simulink model "CCMHfaMotor"  (R2022b) (continued)
== system CCMHfaMotor == (continued)
  HfaActr14BlckSts_HfaActr14BlckSts : Inport  Port=341  OutDataTypeStr=Enum: VentnActr01BlckSts  PortDimensions=1
  HfaActr14BusAdr_HfaActr14BusAdr : Inport  Port=342  OutDataTypeStr=UInt8  PortDimensions=1
  HfaActr14CoilSts_HfaActr14CoilSts : Inport  Port=343  OutDataTypeStr=Enum: VentnMotUrgcUnlck  PortDimensions=1
  HfaActr14DrvDir_HfaActr14DrvDir : Inport  Port=344  OutDataTypeStr=Enum: VentnActr01DrvDir  PortDimensions=1
  HfaActr14ElecErr_HfaActr14ElecErr : Inport  Port=345  OutDataTypeStr=Enum: VentnActr01ElecErr  PortDimensions=1
  HfaActr14Mode_HfaActr14Mode : Inport  Port=346  OutDataTypeStr=Enum: VentnMotMode  PortDimensions=1
  HfaActr14MovDir_HfaActr14MovDir : Inport  Port=347  OutDataTypeStr=Enum: VentnMotDir  PortDimensions=1
  HfaActr14OvrTempErr_HfaActr14OvrTempErr : Inport  Port=348  OutDataTypeStr=Enum: VentnActr01OvrTempErr  PortDimensions=1
  HfaActr14PsonSts_HfaActr14PsonSts : Inport  Port=349  OutDataTypeStr=Enum: VentnMotPsonAvl  PortDimensions=1
  HfaActr14ResetSts_HfaActr14ResetSts : Inport  Port=350  OutDataTypeStr=Enum: VentnActr01ResetSts  PortDimensions=1
  HfaActr14SpclFct_HfaActr14SpclFct : Inport  Port=351  OutDataTypeStr=Enum: VentnActr01SpclFct  PortDimensions=1
  HfaActr14SpdSts_HfaActr14SpdSts : Inport  Port=352  OutDataTypeStr=Enum: VentnActr01SpdSts  PortDimensions=1
  HfaActr14TrqSts_HfaActr14TrqSts : Inport  Port=353  OutDataTypeStr=Enum: VentnMotUrgcUnlck  PortDimensions=1
  HfaActr14UrgcExist_HfaActr14UrgcExist : Inport  Port=354  OutDataTypeStr=Enum: VentnActr01UrgcExist  PortDimensions=1
  HfaActr14UrgcPson_HfaActr14UrgcPson : Inport  Port=355  OutDataTypeStr=Enum: VentnMotUrgcPson  PortDimensions=1
  HfaActr14UrgcSts_HfaActr14UrgcSts : Inport  Port=356  OutDataTypeStr=Enum: VentnMotUrgcUnlck  PortDimensions=1
  HfaActr14VoltErr_HfaActr14VoltErr : Inport  Port=357  OutDataTypeStr=Enum: VentnActr01VoltErr  PortDimensions=1
  HfaActr15ActPson_HfaActr15ActPson : Inport  Port=358  OutDataTypeStr=UInt16  PortDimensions=1
  HfaActr15ActPson_HfaActr15ActPson_IsUpdated : Inport  Port=359  OutDataTypeStr=boolean  PortDimensions=1
  HfaActr15BlckInd_HfaActr15BlckInd : Inport  Port=360  OutDataTypeStr=Enum: VentnActr01BlckInd  PortDimensions=1
  HfaActr15BlckSts_HfaActr15BlckSts : Inport  Port=361  OutDataTypeStr=Enum: VentnActr01BlckSts  PortDimensions=1
  HfaActr15BusAdr_HfaActr15BusAdr : Inport  Port=362  OutDataTypeStr=UInt8  PortDimensions=1
  HfaActr15CoilSts_HfaActr15CoilSts : Inport  Port=363  OutDataTypeStr=Enum: VentnMotUrgcUnlck  PortDimensions=1
  HfaActr15DrvDir_HfaActr15DrvDir : Inport  Port=364  OutDataTypeStr=Enum: VentnActr01DrvDir  PortDimensions=1
  HfaActr15ElecErr_HfaActr15ElecErr : Inport  Port=365  OutDataTypeStr=Enum: VentnActr01ElecErr  PortDimensions=1
  HfaActr15Mode_HfaActr15Mode : Inport  Port=366  OutDataTypeStr=Enum: VentnMotMode  PortDimensions=1
  HfaActr15MovDir_HfaActr15MovDir : Inport  Port=367  OutDataTypeStr=Enum: VentnMotDir  PortDimensions=1
  HfaActr15OvrTempErr_HfaActr15OvrTempErr : Inport  Port=368  OutDataTypeStr=Enum: VentnActr01OvrTempErr  PortDimensions=1
  HfaActr15PsonSts_HfaActr15PsonSts : Inport  Port=369  OutDataTypeStr=Enum: VentnMotPsonAvl  PortDimensions=1
  HfaActr15ResetSts_HfaActr15ResetSts : Inport  Port=370  OutDataTypeStr=Enum: VentnActr01ResetSts  PortDimensions=1
  HfaActr15SpclFct_HfaActr15SpclFct : Inport  Port=371  OutDataTypeStr=Enum: VentnActr01SpclFct  PortDimensions=1
  HfaActr15SpdSts_HfaActr15SpdSts : Inport  Port=372  OutDataTypeStr=Enum: VentnActr01SpdSts  PortDimensions=1
  HfaActr15TrqSts_HfaActr15TrqSts : Inport  Port=373  OutDataTypeStr=Enum: VentnMotUrgcUnlck  PortDimensions=1
  HfaActr15UrgcExist_HfaActr15UrgcExist : Inport  Port=374  OutDataTypeStr=Enum: VentnActr01UrgcExist  PortDimensions=1
  HfaActr15UrgcPson_HfaActr15UrgcPson : Inport  Port=375  OutDataTypeStr=Enum: VentnMotUrgcPson  PortDimensions=1
  HfaActr15UrgcSts_HfaActr15UrgcSts : Inport  Port=376  OutDataTypeStr=Enum: VentnMotUrgcUnlck  PortDimensions=1
  HfaActr15VoltErr_HfaActr15VoltErr : Inport  Port=377  OutDataTypeStr=Enum: VentnActr01VoltErr  PortDimensions=1
  HvacCfgForClimaCtrl_HvacCfgForAQSTypes : Inport  Port=378  OutDataTypeStr=UInt8  PortDimensions=1
  HvacCfgForClimaCtrl_HvacCfgForHvacLowSideRETXVESts : Inport  Port=379  OutDataTypeStr=UInt8  PortDimensions=1
  HvacCfgForClimaCtrl_HvacCfgForHvacReBlowerType : Inport  Port=380  OutDataTypeStr=UInt8  PortDimensions=1
  HvacCfgForClimaCtrl_HvacCfgForHvacReVentPstn : Inport  Port=381  OutDataTypeStr=UInt8  PortDimensions=1
  HvacCfgForClimaCtrl_HvacCfgForHvacSnsrRCmptmtESts : Inport  Port=382  OutDataTypeStr=UInt8  PortDimensions=1
  HvacCfgForClimaCtrl_HvacCfgForHvacSnsrReHexESts : Inport  Port=383  OutDataTypeStr=UInt8  PortDimensions=1
  Lin1ActvReady_Lin1ActvReady : Inport  Port=384  OutDataTypeStr=UInt8  PortDimensions=1
  Lin2ActvReady_Lin2ActvReady : Inport  Port=385  OutDataTypeStr=UInt8  PortDimensions=1
  ReHvacActrActPson_ReHvacActrActPson : Inport  Port=386  OutDataTypeStr=UInt16  PortDimensions=1
  ReHvacActrActPson_ReHvacActrActPson_IsUpdated : Inport  Port=387  OutDataTypeStr=boolean  PortDimensions=1
  ReHvacActrBlckInd_ReHvacActrBlckInd : Inport  Port=388  OutDataTypeStr=Enum: VentnActr01BlckInd  PortDimensions=1
  ReHvacActrBlckSts_ReHvacActrBlckSts : Inport  Port=389  OutDataTypeStr=Enum: VentnActr01BlckSts  PortDimensions=1
  ReHvacActrBusAdr_ReHvacActrBusAdr : Inport  Port=390  OutDataTypeStr=UInt8  PortDimensions=1
  ReHvacActrCoilSts_ReHvacActrCoilSts : Inport  Port=391  OutDataTypeStr=Enum: VentnMotUrgcUnlck  PortDimensions=1
  ReHvacActrDrvDir_ReHvacActrDrvDir : Inport  Port=392  OutDataTypeStr=Enum: VentnActr01DrvDir  PortDimensions=1
  ReHvacActrElecErr_ReHvacActrElecErr : Inport  Port=393  OutDataTypeStr=Enum: VentnActr01ElecErr  PortDimensions=1
  ReHvacActrMode_ReHvacActrMode : Inport  Port=394  OutDataTypeStr=Enum: VentnMotMode  PortDimensions=1
  ReHvacActrMovDir_ReHvacActrMovDir : Inport  Port=395  OutDataTypeStr=Enum: VentnMotDir  PortDimensions=1
  ReHvacActrOvrTempErr_ReHvacActrOvrTempErr : Inport  Port=396  OutDataTypeStr=Enum: VentnActr01OvrTempErr  PortDimensions=1
  ReHvacActrPsonSts_ReHvacActrPsonSts : Inport  Port=397  OutDataTypeStr=Enum: VentnMotPsonAvl  PortDimensions=1
  ReHvacActrResetSts_ReHvacActrResetSts : Inport  Port=398  OutDataTypeStr=Enum: VentnActr01ResetSts  PortDimensions=1
  ReHvacActrSpclFct_ReHvacActrSpclFct : Inport  Port=399  OutDataTypeStr=Enum: VentnActr01SpclFct  PortDimensions=1
  ReHvacActrSpdSts_ReHvacActrSpdSts : Inport  Port=400  OutDataTypeStr=Enum: VentnActr01SpdSts  PortDimensions=1
  ReHvacActrTrqSts_ReHvacActrTrqSts : Inport  Port=401  OutDataTypeStr=Enum: VentnMotUrgcUnlck  PortDimensions=1
  ReHvacActrUrgcExist_ReHvacActrUrgcExist : Inport  Port=402  OutDataTypeStr=Enum: VentnActr01UrgcExist  PortDimensions=1
  ReHvacActrUrgcPson_ReHvacActrUrgcPson : Inport  Port=403  OutDataTypeStr=Enum: VentnMotUrgcPson  PortDimensions=1
  ReHvacActrUrgcSts_ReHvacActrUrgcSts : Inport  Port=404  OutDataTypeStr=Enum: VentnMotUrgcUnlck  PortDimensions=1
  ReHvacActrVoltErr_ReHvacActrVoltErr : Inport  Port=405  OutDataTypeStr=Enum: VentnActr01VoltErr  PortDimensions=1
  SysServCarCfgSts_SysServCCPBGMNum : Inport  Port=406  OutDataTypeStr=UInt8  PortDimensions=1
  SysServCarCfgSts_SysServCCPLin2AQSSts : Inport  Port=407  OutDataTypeStr=Boolean  PortDimensions=1
  SysServCarCfgSts_SysServDUBlowerPwmSignTypeSts : Inport  Port=408  OutDataTypeStr=UInt8  PortDimensions=1
  SysServCarCfgSts_SysServDUDCFLMdeSts : Inport  Port=409  OutDataTypeStr=UInt8  PortDimensions=1
  SysServCarCfgSts_SysServDULin4HFAFStsH : Inport  Port=410  OutDataTypeStr=UInt32  PortDimensions=1
  SysServCarCfgSts_SysServDUReBlowerPwmSignSts : Inport  Port=411  OutDataTypeStr=UInt8  PortDimensions=1
  SysServCarCfgSts_SysServDUReBlowerPwmSts : Inport  Port=412  OutDataTypeStr=UInt8  PortDimensions=1
  SysServCarCfgSts_SysServFloatingMotorSts : Inport  Port=413  OutDataTypeStr=UInt8  PortDimensions=1
  SysServCarCfgSts_SysServFrntHEXSnsrPosn : Inport  Port=414  OutDataTypeStr=UInt8  PortDimensions=1
  SysServCarCfgSts_SysServLowSideRETXVSts : Inport  Port=415  OutDataTypeStr=UInt8  PortDimensions=1
  SysServCarCfgSts_SysServReHEXSnsrPosn : Inport  Port=416  OutDataTypeStr=UInt8  PortDimensions=1
  SysServCarCfgSts_SysServRHFASts : Inport  Port=417  OutDataTypeStr=UInt8  PortDimensions=1
  SysServCarCfgSts_SysServSnsrForFCmptmtTType : Inport  Port=418  OutDataTypeStr=UInt8  PortDimensions=1
  WtrActrLeActPson_WtrActrLeActPson : Inport  Port=419  OutDataTypeStr=UInt16  PortDimensions=1
  WtrActrLeBlckInd_WtrActrLeBlckInd : Inport  Port=420  OutDataTypeStr=Enum: VentnActr01BlckInd  PortDimensions=1
  WtrActrLeBlckSts_WtrActrLeBlckSts : Inport  Port=421  OutDataTypeStr=Enum: VentnActr01BlckSts  PortDimensions=1
  WtrActrLeBusAdr_WtrActrLeBusAdr : Inport  Port=422  OutDataTypeStr=UInt8  PortDimensions=1
  WtrActrLeCoilSts_WtrActrLeCoilSts : Inport  Port=423  OutDataTypeStr=Enum: VentnMotUrgcUnlck  PortDimensions=1
  WtrActrLeDir_WtrActrLeDir : Inport  Port=424  OutDataTypeStr=Enum: VentnActr01DrvDir  PortDimensions=1
  WtrActrLeElecErr_WtrActrLeElecErr : Inport  Port=425  OutDataTypeStr=Enum: VentnActr01ElecErr  PortDimensions=1
  WtrActrLeMode_WtrActrLeMode : Inport  Port=426  OutDataTypeStr=Enum: VentnMotMode  PortDimensions=1
  WtrActrLeMovDir_WtrActrLeMovDir : Inport  Port=427  OutDataTypeStr=Enum: VentnMotDir  PortDimensions=1
  WtrActrLeOvrTempErr_WtrActrLeOvrTempErr : Inport  Port=428  OutDataTypeStr=Enum: VentnActr01OvrTempErr  PortDimensions=1
  WtrActrLePsonSts_WtrActrLePsonSts : Inport  Port=429  OutDataTypeStr=Enum: VentnMotPsonAvl  PortDimensions=1
  WtrActrLeResetSts_WtrActrLeResetSts : Inport  Port=430  OutDataTypeStr=Enum: VentnActr01ResetSts  PortDimensions=1
  WtrActrLeSpclFct_WtrActrLeSpclFct : Inport  Port=431  OutDataTypeStr=Enum: VentnActr01SpclFct  PortDimensions=1
  WtrActrLeSpdSts_WtrActrLeSpdSts : Inport  Port=432  OutDataTypeStr=Enum: VentnActr01SpdSts  PortDimensions=1
  WtrActrLeTrqSts_WtrActrLeTrqSts : Inport  Port=433  OutDataTypeStr=Enum: VentnMotUrgcUnlck  PortDimensions=1
  WtrActrLeUrgcExist_WtrActrLeUrgcExist : Inport  Port=434  OutDataTypeStr=Enum: VentnActr01UrgcExist  PortDimensions=1
  WtrActrLeUrgcPson_WtrActrLeUrgcPson : Inport  Port=435  OutDataTypeStr=Enum: VentnMotUrgcPson  PortDimensions=1
  WtrActrLeUrgcSts_WtrActrLeUrgcSts : Inport  Port=436  OutDataTypeStr=Enum: VentnMotUrgcUnlck  PortDimensions=1
  WtrActrLeVoltErr_WtrActrLeVoltErr : Inport  Port=437  OutDataTypeStr=Enum: VentnActr01VoltErr  PortDimensions=1
  WtrActrRiActPson_WtrActrRiActPson : Inport  Port=438  OutDataTypeStr=UInt16  PortDimensions=1
  WtrActrRiBlckInd_WtrActrRiBlckInd : Inport  Port=439  OutDataTypeStr=Enum: VentnActr01BlckInd  PortDimensions=1
  WtrActrRiBlckSts_WtrActrRiBlckSts : Inport  Port=440  OutDataTypeStr=Enum: VentnActr01BlckSts  PortDimensions=1
  WtrActrRiBusAdr_WtrActrRiBusAdr : Inport  Port=441  OutDataTypeStr=UInt8  PortDimensions=1
  WtrActrRiCoilSts_WtrActrRiCoilSts : Inport  Port=442  OutDataTypeStr=Enum: VentnMotUrgcUnlck  PortDimensions=1
  WtrActrRiDir_WtrActrRiDir : Inport  Port=443  OutDataTypeStr=Enum: VentnActr01DrvDir  PortDimensions=1
  WtrActrRiElecErr_WtrActrRiElecErr : Inport  Port=444  OutDataTypeStr=Enum: VentnActr01ElecErr  PortDimensions=1
  WtrActrRiMode_WtrActrRiMode : Inport  Port=445  OutDataTypeStr=Enum: VentnMotMode  PortDimensions=1
  WtrActrRiMovDir_WtrActrRiMovDir : Inport  Port=446  OutDataTypeStr=Enum: VentnMotDir  PortDimensions=1
  WtrActrRiOvrTempErr_WtrActrRiOvrTempErr : Inport  Port=447  OutDataTypeStr=Enum: VentnActr01OvrTempErr  PortDimensions=1
  WtrActrRiPsonSts_WtrActrRiPsonSts : Inport  Port=448  OutDataTypeStr=Enum: VentnMotPsonAvl  PortDimensions=1
  WtrActrRiResetSts_WtrActrRiResetSts : Inport  Port=449  OutDataTypeStr=Enum: VentnActr01ResetSts  PortDimensions=1
  WtrActrRiSpclFct_WtrActrRiSpclFct : Inport  Port=450  OutDataTypeStr=Enum: VentnActr01SpclFct  PortDimensions=1
  WtrActrRiSpdSts_WtrActrRiSpdSts : Inport  Port=451  OutDataTypeStr=Enum: VentnActr01SpdSts  PortDimensions=1
  WtrActrRiTrqSts_WtrActrRiTrqSts : Inport  Port=452  OutDataTypeStr=Enum: VentnMotUrgcUnlck  PortDimensions=1
  WtrActrRiUrgcExist_WtrActrRiUrgcExist : Inport  Port=453  OutDataTypeStr=Enum: VentnActr01UrgcExist  PortDimensions=1
  WtrActrRiUrgcPson_WtrActrRiUrgcPson : Inport  Port=454  OutDataTypeStr=Enum: VentnMotUrgcPson  PortDimensions=1
  WtrActrRiUrgcSts_WtrActrRiUrgcSts : Inport  Port=455  OutDataTypeStr=Enum: VentnMotUrgcUnlck  PortDimensions=1
  WtrActrRiVoltErr_WtrActrRiVoltErr : Inport  Port=456  OutDataTypeStr=Enum: VentnActr01VoltErr  PortDimensions=1
  BswLifeCycle_LifeCycle : Inport  Port=457  OutDataTypeStr=Enum: CCMLifeCycleModeGroup  PortDimensions=1
  CCMHfaMotor_HfaMotMode_sys : Subsystem
  CCMHfaMotor_ReHvacMotMode_sys : Subsystem
  CCMHfaMotor_WtrMotMode_sys : Subsystem
  HfaCaliService : Subsystem
  HfaDtcService : Subsystem
  HfaIOCtrlService : Subsystem
  HfaMotor_50msRunnable_sys : Subsystem
  HfaMotor_Init : Subsystem
  HfaMotor_Runnable_sys : Subsystem
  HfaNvmService : Subsystem
  HfaParaService : Subsystem
  HfaRIDService : Subsystem
  NvMBlockHfaMtrCalData : DataStoreMemory  DataStoreName=NvMBlockHfaMtrCalData  InitialValue=NvMBlockHfaMtrCalDataInit  ShowAdditionalParam=off  Dimensions=200  StateMustResolveToSignalObject=on
  ActCfrAddrFinalAddr_ActCfrAddrFinalAddr : Outport  OutDataTypeStr=UInt8  PortDimensions=1
  ActCfrAddrGroup_ActCfrAddrGroup : Outport  Port=2  OutDataTypeStr=Enum: ActCfrGroup  PortDimensions=1
  ActCfrAddrItem_ActCfrAddrItem : Outport  Port=3  OutDataTypeStr=Uint5  PortDimensions=1
  ActCfrIniCPos_ActCfrIniCPos : Outport  Port=4  OutDataTypeStr=UInt15  PortDimensions=1
  ActCfrIniGroup_ActCfrIniGroup : Outport  Port=5  OutDataTypeStr=Enum: ActCfrGroup  PortDimensions=1
  ActCfrIniItem_ActCfrIniItem : Outport  Port=6  OutDataTypeStr=Uint5  PortDimensions=1
  ActCfrIniResvd_ActCfrIniResvd : Outport  Port=7  OutDataTypeStr=UInt8  PortDimensions=1
  ActCfrModGroup_ActCfrModGroup : Outport  Port=8  OutDataTypeStr=Enum: ActCfrGroup  PortDimensions=1
  ActCfrModItem_ActCfrModItem : Outport  Port=9  OutDataTypeStr=Uint5  PortDimensions=1
  ActCfrModNewMod_ActCfrModNewMod : Outport  Port=10  OutDataTypeStr=Enum: ActCfrMod  PortDimensions=1
  ActCfrModResvd1_ActCfrModResvd1 : Outport  Port=11  OutDataTypeStr=UInt8  PortDimensions=1
  ActCfrModResvd2_ActCfrModResvd2 : Outport  Port=12  OutDataTypeStr=UInt8  PortDimensions=1
  ActCfrPrgDiret_ActCfrPrgDiret : Outport  Port=13  OutDataTypeStr=Enum: ActCfrPrgDir  PortDimensions=1
  ActCfrPrgGroup_ActCfrPrgGroup : Outport  Port=14  OutDataTypeStr=Enum: ActCfrGroup  PortDimensions=1
  ActCfrPrgItem_ActCfrPrgItem : Outport  Port=15  OutDataTypeStr=Uint5  PortDimensions=1
  ActCfrPrgPrgGroup_ActCfrPrgPrgGroup : Outport  Port=16  OutDataTypeStr=Enum: ActCfrGroup  PortDimensions=1
  ActCfrPrgPrgItem_ActCfrPrgPrgItem : Outport  Port=17  OutDataTypeStr=Uint5  PortDimensions=1
  ActCfrPrgRef_ActCfrPrgRef : Outport  Port=18  OutDataTypeStr=UInt8  PortDimensions=1
  ActCfrPrgSafety_ActCfrPrgSafety : Outport  Port=19  OutDataTypeStr=Enum: ActCfrPrgSafty  PortDimensions=1
  ActCfrPrgSafetyEnable_ActCfrPrgSafetyEnable : Outport  Port=20  OutDataTypeStr=Enum: ActvInActv  PortDimensions=1
  ActCfrSetAutoSpd_ActCfrSetAutoSpd : Outport  Port=21  OutDataTypeStr=Enum: OnOff1  PortDimensions=1
  ActCfrSetFPos_ActCfrSetFPos : Outport  Port=22  OutDataTypeStr=UInt15  PortDimensions=1
  ActCfrSetGroup_ActCfrSetGroup : Outport  Port=23  OutDataTypeStr=Enum: ActCfrGroup  PortDimensions=1
  ActCfrSetItem_ActCfrSetItem : Outport  Port=24  OutDataTypeStr=Uint5  PortDimensions=1
  ActCfrSetLowNoise_ActCfrSetLowNoise : Outport  Port=25  OutDataTypeStr=Enum: OnOff2  PortDimensions=1
  ActCfrSetSpd_ActCfrSetSpd : Outport  Port=26  OutDataTypeStr=Enum: SetSpdMode  PortDimensions=1
  ActCfrSetStallDet_ActCfrSetStallDet : Outport  Port=27  OutDataTypeStr=Enum: OnOff2  PortDimensions=1
  ActCfrSetStopPhs_ActCfrSetStopPhs : Outport  Port=28  OutMin=0  OutMax=3  OutDataTypeStr=UInt2  PortDimensions=1
  ActDfrIdeGroup_ActDfrIdeGroup : Outport  Port=29  OutDataTypeStr=Enum: ActCfrGroup  PortDimensions=1
  ActDfrIdeItem_ActDfrIdeItem : Outport  Port=30  OutDataTypeStr=Uint5  PortDimensions=1
  ActDfrIdeResvd_ActDfrIdeResvd : Outport  Port=31  OutDataTypeStr=UInt8  PortDimensions=1
  ActDfrPrgGroup_ActDfrPrgGroup : Outport  Port=32  OutDataTypeStr=Enum: ActCfrGroup  PortDimensions=1
  ActDfrPrgItem_ActDfrPrgItem : Outport  Port=33  OutDataTypeStr=Uint5  PortDimensions=1
  ActDfrPrgResvd_ActDfrPrgResvd : Outport  Port=34  OutDataTypeStr=UInt8  PortDimensions=1
  ActDfrStaGroup_ActDfrStaGroup : Outport  Port=35  OutDataTypeStr=Enum: ActCfrGroup  PortDimensions=1
  ActDfrStaItem_ActDfrStaItem : Outport  Port=36  OutDataTypeStr=Uint5  PortDimensions=1
  ActDfrStaResvd_ActDfrStaResvd : Outport  Port=37  OutDataTypeStr=UInt8  PortDimensions=1
  CcmHfaMasterReqB0Lin4_CcmHfaMasterReqB0Lin4 : Outport  Port=38  OutDataTypeStr=UInt8  PortDimensions=1
  CcmHfaMasterReqB1Lin4_CcmHfaMasterReqB1Lin4 : Outport  Port=39  OutDataTypeStr=UInt8  PortDimensions=1
  CcmHfaMasterReqB2Lin4_CcmHfaMasterReqB2Lin4 : Outport  Port=40  OutDataTypeStr=UInt8  PortDimensions=1
  CcmHfaMasterReqB3Lin4_CcmHfaMasterReqB3Lin4 : Outport  Port=41  OutDataTypeStr=UInt8  PortDimensions=1
  CcmHfaMasterReqB4Lin4_CcmHfaMasterReqB3Lin4 : Outport  Port=42  OutDataTypeStr=UInt8  PortDimensions=1
  CcmHfaMasterReqB5Lin4_CcmHfaMasterReqB5Lin4 : Outport  Port=43  OutDataTypeStr=UInt8  PortDimensions=1
  CcmHfaMasterReqB6Lin4_CcmHfaMasterReqB6Lin4 : Outport  Port=44  OutDataTypeStr=UInt8  PortDimensions=1
  CcmHfaMasterReqB7Lin4_CcmHfaMasterReqB7Lin4 : Outport  Port=45  OutDataTypeStr=UInt8  PortDimensions=1
  Lin1HfaActvReq_Lin1HfaActvReq : Outport  Port=46  OutDataTypeStr=UInt8  PortDimensions=1
  ReHvacMotAdr_ReHvacMotAdr : Outport  Port=47  OutDataTypeStr=UInt8  PortDimensions=1
  ReHvacMotBlockCmd_ReHvacMotBlockCmd : Outport  Port=48  OutDataTypeStr=Enum: VentnMotBlockCmd  PortDimensions=1
  ReHvacMotClearReq_ReHvacMotClearReq : Outport  Port=49  OutMin=0  OutMax=15  OutDataTypeStr=UInt4  PortDimensions=1
  ReHvacMotCoilPwrOn_ReHvacMotCoilPwrOn : Outport  Port=50  OutDataTypeStr=Enum: VentnMotUrgcUnlck  PortDimensions=1
  ReHvacMotDir_ReHvacMotDir : Outport  Port=51  OutDataTypeStr=Enum: VentnMotDir  PortDimensions=1
  ReHvacMotMode_ReHvacMotMode : Outport  Port=52  OutDataTypeStr=Enum: VentnMotMode  PortDimensions=1
  ReHvacMotPsonAvl_ReHvacMotPsonAvl : Outport  Port=53  OutDataTypeStr=Enum: VentnMotPsonAvl  PortDimensions=1
  ReHvacMotSaveData_ReHvacMotSaveData : Outport  Port=54  OutDataTypeStr=Enum: VentnMotSaveData  PortDimensions=1
  ReHvacMotSpdSet_ReHvacMotSpdSet : Outport  Port=55  OutDataTypeStr=Enum: VentnMotSpdSet  PortDimensions=1
  ReHvacMotStrtPson_ReHvacMotStrtPson : Outport  Port=56  OutDataTypeStr=UInt16  PortDimensions=1
  ReHvacMotTargtPson_ReHvacMotTargtPson : Outport  Port=57  OutDataTypeStr=UInt16  PortDimensions=1
  ReHvacMotUrgcPson_ReHvacMotUrgcPson : Outport  Port=58  OutDataTypeStr=Enum: VentnMotUrgcPson  PortDimensions=1
  ReHvacMotUrgcUnlck_ReHvacMotUrgcUnlck : Outport  Port=59  OutDataTypeStr=Enum: VentnMotUrgcUnlck  PortDimensions=1
  RHFAScheTableReq_Val : Outport  Port=60  OutDataTypeStr=UInt8  PortDimensions=1
  WtrMotAdr_WtrMotAdr : Outport  Port=61  OutDataTypeStr=UInt8  PortDimensions=1
  WtrMotBlockCmd_WtrMotBlockCmd : Outport  Port=62  OutDataTypeStr=Enum: VentnMotBlockCmd  PortDimensions=1
  WtrMotClearReq_WtrMotClearReq : Outport  Port=63  OutMin=0  OutMax=15  OutDataTypeStr=UInt4  PortDimensions=1
  WtrMotCoilPwrOn_WtrMotCoilPwrOn : Outport  Port=64  OutDataTypeStr=Enum: VentnMotUrgcUnlck  PortDimensions=1
  WtrMotDir_WtrMotDir : Outport  Port=65  OutDataTypeStr=Enum: VentnMotDir  PortDimensions=1
  WtrMotMode_WtrMotMode : Outport  Port=66  OutDataTypeStr=Enum: VentnMotMode  PortDimensions=1
  WtrMotPsonAvl_WtrMotPsonAvl : Outport  Port=67  OutDataTypeStr=Enum: VentnMotPsonAvl  PortDimensions=1
  WtrMotSaveData_WtrMotSaveData : Outport  Port=68  OutDataTypeStr=Enum: VentnMotSaveData  PortDimensions=1
  WtrMotSpdSet_WtrMotSpdSet : Outport  Port=69  OutDataTypeStr=Enum: VentnMotSpdSet  PortDimensions=1
  WtrMotStrtPson_WtrMotStrtPson : Outport  Port=70  OutDataTypeStr=UInt16  PortDimensions=1
  WtrMotTargtPson_WtrMotTargtPson : Outport  Port=71  OutDataTypeStr=UInt16  PortDimensions=1
  WtrMotUrgcPson_WtrMotUrgcPson : Outport  Port=72  OutDataTypeStr=Enum: VentnMotUrgcPson  PortDimensions=1
  WtrMotUrgcUnlck_WtrMotUrgcUnlck : Outport  Port=73  OutDataTypeStr=Enum: VentnMotUrgcUnlck  PortDimensions=1
  HfaMotAdr_HfaMotAdr : Outport  Port=74  OutDataTypeStr=UInt8  PortDimensions=1
  HfaMotBlockCmd_HfaMotBlockCmd : Outport  Port=75  OutDataTypeStr=Enum: VentnMotBlockCmd  PortDimensions=1
  HfaMotClearReq_HfaMotClearReq : Outport  Port=76  OutMin=0  OutMax=15  OutDataTypeStr=UInt4  PortDimensions=1
  HfaMotCoilPwrOn_HfaMotCoilPwrOn : Outport  Port=77  OutDataTypeStr=Enum: VentnMotUrgcUnlck  PortDimensions=1
  HfaMotDir_HfaMotDir : Outport  Port=78  OutDataTypeStr=Enum: VentnMotDir  PortDimensions=1
  HfaMotMode_HfaMotMode : Outport  Port=79  OutDataTypeStr=Enum: VentnMotMode  PortDimensions=1
  HfaMotPsonAvl_HfaMotPsonAvl : Outport  Port=80  OutDataTypeStr=Enum: VentnMotPsonAvl  PortDimensions=1
  HfaMotSaveData_HfaMotSaveData : Outport  Port=81  OutDataTypeStr=Enum: VentnMotSaveData  PortDimensions=1
  HfaMotSpdSet_HfaMotSpdSet : Outport  Port=82  OutDataTypeStr=Enum: VentnMotSpdSet  PortDimensions=1
  HfaMotStrtPson_HfaMotStrtPson : Outport  Port=83  OutDataTypeStr=UInt16  PortDimensions=1
  HfaMotTargtPson_HfaMotTargtPson : Outport  Port=84  OutDataTypeStr=UInt16  PortDimensions=1
  HfaMotUrgcPson_HfaMotUrgcPson : Outport  Port=85  OutDataTypeStr=Enum: VentnMotUrgcPson  PortDimensions=1
  HfaMotUrgcUnlck_HfaMotUrgcUnlck : Outport  Port=86  OutDataTypeStr=Enum: VentnMotUrgcUnlck  PortDimensions=1
  wires:
  AcRfrPrgSafety_AcRfrPrgSafety/1 -> HfaMotor_Runnable_sys/1(AcRfrPrgSafety_AcRfrPrgSafety_read)
  ActRfrIdeGroup_ActRfrIdeGroup/1 -> HfaMotor_Runnable_sys/2(ActRfrIdeGroup_ActRfrIdeGroup_read)
  ActRfrIdeItem_ActRfrIdeItem/1 -> HfaMotor_Runnable_sys/3(ActRfrIdeItem_ActRfrIdeItem_read)
  ActRfrIdePN_ActRfrIdePN/1 -> HfaMotor_Runnable_sys/4(ActRfrIdePN_ActRfrIdePN_read)
  ActRfrIdeSupplier_ActRfrIdeSupplier/1 -> HfaMotor_Runnable_sys/5(ActRfrIdeSupplier_ActRfrIdeSupplier_read)
  ActRfrIdeVers_ActRfrIdeVers/1 -> HfaMotor_Runnable_sys/6(ActRfrIdeVers_ActRfrIdeVers_read)
  ActRfrPrgDiret_ActRfrPrgDiret/1 -> HfaMotor_Runnable_sys/7(ActRfrPrgDiret_ActRfrPrgDiret_read)
  ActRfrPrgGroup_ActRfrPrgGroup/1 -> HfaMotor_Runnable_sys/8(ActRfrPrgGroup_ActRfrPrgGroup_read)
  ActRfrPrgItem_ActRfrPrgItem/1 -> HfaMotor_Runnable_sys/9(ActRfrPrgItem_ActRfrPrgItem_read)
  ActRfrPrgPrgGroup_ActRfrPrgPrgGroup/1 -> HfaMotor_Runnable_sys/10(ActRfrPrgPrgGroup_ActRfrPrgPrgGroup_read)
  ActRfrPrgPrgItem_ActRfrPrgPrgItem/1 -> HfaMotor_Runnable_sys/11(ActRfrPrgPrgItem_ActRfrPrgPrgItem_read)
  ActRfrPrgRef_ActRfrPrgRef/1 -> HfaMotor_Runnable_sys/12(ActRfrPrgRef_ActRfrPrgRef_read)
  ActRfrPrgSafetyEnable_ActRfrPrgSafetyEnable/1 -> HfaMotor_Runnable_sys/13(ActRfrPrgSafetyEnable_ActRfrPrgSafetyEnable_read)
  ActRfrStaCPos_ActRfrStaCPos/1 -> HfaMotor_Runnable_sys/14(ActRfrStaCPos_ActRfrStaCPos_read)
  ActRfrStaEmgyRun_ActRfrStaEmgyRun/1 -> HfaMotor_Runnable_sys/15(ActRfrStaEmgyRun_ActRfrStaEmgyRun_read)
  ActRfrStaErr_ActRfrStaErr/1 -> HfaMotor_Runnable_sys/16(ActRfrStaErr_ActRfrStaErr_read)
  ActRfrStaGroup_ActRfrStaGroup/1 -> HfaMotor_Runnable_sys/17(ActRfrStaGroup_ActRfrStaGroup_read)
  ActRfrStaItem_ActRfrStaItem/1 -> HfaMotor_Runnable_sys/18(ActRfrStaItem_ActRfrStaItem_read)
  ActRfrStaMod_ActRfrStaMod/1 -> HfaMotor_Runnable_sys/19(ActRfrStaMod_ActRfrStaMod_read)
  ActRfrStaReset_ActRfrStaReset/1 -> HfaMotor_Runnable_sys/20(ActRfrStaReset_ActRfrStaReset_read)
  ActRfrStaStd_ActRfrStaStd/1 -> HfaMotor_Runnable_sys/21(ActRfrStaStd_ActRfrStaStd_read)
  BatSysBtrMSts_BatSysBtrMSts/1 -> HfaMotor_Runnable_sys/22(BatSysBtrMSts_BatSysBtrMSts_read)
  HfaCcmSlaveRespB0Lin4_HfaCcmSlaveRespB0Lin4/1 -> HfaMotor_Runnable_sys/23(HfaCcmSlaveRespB0Lin4_HfaCcmSlaveRespB0Lin4_read)
  HfaCcmSlaveRespB1Lin4_HfaCcmSlaveRespB1Lin4/1 -> HfaMotor_Runnable_sys/24(HfaCcmSlaveRespB1Lin4_HfaCcmSlaveRespB1Lin4_read)
  HfaCcmSlaveRespB2Lin4_HfaCcmSlaveRespB2Lin4/1 -> HfaMotor_Runnable_sys/25(HfaCcmSlaveRespB2Lin4_HfaCcmSlaveRespB2Lin4_read)
  HfaCcmSlaveRespB3Lin4_HfaCcmSlaveRespB3Lin4/1 -> HfaMotor_Runnable_sys/26(HfaCcmSlaveRespB3Lin4_HfaCcmSlaveRespB3Lin4_read)
  HfaCcmSlaveRespB4Lin4_HfaCcmSlaveRespB4Lin4/1 -> HfaMotor_Runnable_sys/27(HfaCcmSlaveRespB4Lin4_HfaCcmSlaveRespB4Lin4_read)
  HfaCcmSlaveRespB5Lin4_HfaCcmSlaveRespB5Lin4/1 -> HfaMotor_Runnable_sys/28(HfaCcmSlaveRespB5Lin4_HfaCcmSlaveRespB5Lin4_read)
  HfaCcmSlaveRespB6Lin4_HfaCcmSlaveRespB6Lin4/1 -> HfaMotor_Runnable_sys/29(HfaCcmSlaveRespB6Lin4_HfaCcmSlaveRespB6Lin4_read)
  HfaCcmSlaveRespB7Lin4_HfaCcmSlaveRespB7Lin4/1 -> HfaMotor_Runnable_sys/30(HfaCcmSlaveRespB7Lin4_HfaCcmSlaveRespB7Lin4_read)
  HmiElecAirSwngReq_HmiElecAirSwngReq/1 -> HfaMotor_Runnable_sys/31(HmiElecAirSwngReq_HmiElecAirSwngReq_read)
  HvacCfgForClimaCtrl_HvacCfgForHvacFrontBlowerType/1 -> HfaMotor_Runnable_sys/32(HvacCfgForClimaCtrl_HvacCfgForHvacFrontBlowerType_read)
  HvacCfgForClimaCtrl_HvacCfgForHvacMiscExistSts/1 -> HfaMotor_Runnable_sys/33(HvacCfgForClimaCtrl_HvacCfgForHvacMiscExistSts_read)
  HvacCfgForClimaCtrl_HvacCfgForHvacMotorExistSts/1 -> HfaMotor_Runnable_sys/34(HvacCfgForClimaCtrl_HvacCfgForHvacMotorExistSts_read)
  HvacCfgForClimaCtrl_HvacCfgForHvacSensorExistSts/1 -> HfaMotor_Runnable_sys/35(HvacCfgForClimaCtrl_HvacCfgForHvacSensorExistSts_read)
  SysServCarCfgSts_SysServCarID/1 -> HfaMotor_Runnable_sys/38(SysServCarCfgSts_SysServCarID_read)
  SysServCarCfgSts_SysServCCPAQSSts/1 -> HfaMotor_Runnable_sys/39(SysServCarCfgSts_SysServCCPAQSSts_read)
  SysServCarCfgSts_SysServCCPHEXSensorSts/1 -> HfaMotor_Runnable_sys/40(SysServCarCfgSts_SysServCCPHEXSensorSts_read)
  SysServCarCfgSts_SysServCCPLin2AFUSts/1 -> HfaMotor_Runnable_sys/41(SysServCarCfgSts_SysServCCPLin2AFUSts_read)
  SysServCarCfgSts_SysServCCPLin2AIUSts/1 -> HfaMotor_Runnable_sys/42(SysServCarCfgSts_SysServCCPLin2AIUSts_read)
  SysServCarCfgSts_SysServCCPLin2CDSSts/1 -> HfaMotor_Runnable_sys/43(SysServCarCfgSts_SysServCCPLin2CDSSts_read)
  SysServCarCfgSts_SysServCCPLin2PMSISts/1 -> HfaMotor_Runnable_sys/44(SysServCarCfgSts_SysServCCPLin2PMSISts_read)
  SysServCarCfgSts_SysServCCPLin3VGASts/1 -> HfaMotor_Runnable_sys/45(SysServCarCfgSts_SysServCCPLin3VGASts_read)
  SysServCarCfgSts_SysServDUAirVentSnrSts/1 -> HfaMotor_Runnable_sys/46(SysServCarCfgSts_SysServDUAirVentSnrSts_read)
  SysServCarCfgSts_SysServDUBlowerPwmSignSts/1 -> HfaMotor_Runnable_sys/47(SysServCarCfgSts_SysServDUBlowerPwmSignSts_read)
  SysServCarCfgSts_SysServDUBlowerPwmSts/1 -> HfaMotor_Runnable_sys/48(SysServCarCfgSts_SysServDUBlowerPwmSts_read)
  SysServCarCfgSts_SysServDUDCDefrostSts/1 -> HfaMotor_Runnable_sys/49(SysServCarCfgSts_SysServDUDCDefrostSts_read)
  SysServCarCfgSts_SysServDUDCModeSts/1 -> HfaMotor_Runnable_sys/50(SysServCarCfgSts_SysServDUDCModeSts_read)
  SysServCarCfgSts_SysServDUDCRecSts/1 -> HfaMotor_Runnable_sys/51(SysServCarCfgSts_SysServDUDCRecSts_read)
  SysServCarCfgSts_SysServDUDCSModeSts/1 -> HfaMotor_Runnable_sys/52(SysServCarCfgSts_SysServDUDCSModeSts_read)
  SysServCarCfgSts_SysServDUDCSTLSts/1 -> HfaMotor_Runnable_sys/53(SysServCarCfgSts_SysServDUDCSTLSts_read)
  SysServCarCfgSts_SysServDUDCTLSts/1 -> HfaMotor_Runnable_sys/54(SysServCarCfgSts_SysServDUDCTLSts_read)
  SysServCarCfgSts_SysServDUDCTRSts/1 -> HfaMotor_Runnable_sys/55(SysServCarCfgSts_SysServDUDCTRSts_read)
  SysServCarCfgSts_SysServDUFLFootSts/1 -> HfaMotor_Runnable_sys/56(SysServCarCfgSts_SysServDUFLFootSts_read)
  SysServCarCfgSts_SysServDUFLVentSts/1 -> HfaMotor_Runnable_sys/57(SysServCarCfgSts_SysServDUFLVentSts_read)
  SysServCarCfgSts_SysServDUFRFootSts/1 -> HfaMotor_Runnable_sys/58(SysServCarCfgSts_SysServDUFRFootSts_read)
  SysServCarCfgSts_SysServDUFRVentSts/1 -> HfaMotor_Runnable_sys/59(SysServCarCfgSts_SysServDUFRVentSts_read)
  SysServCarCfgSts_SysServDUIncarMotorSts/1 -> HfaMotor_Runnable_sys/60(SysServCarCfgSts_SysServDUIncarMotorSts_read)
  SysServCarCfgSts_SysServDULin1HBMFSts/1 -> HfaMotor_Runnable_sys/61(SysServCarCfgSts_SysServDULin1HBMFSts_read)
  SysServCarCfgSts_SysServDULin4HFAFSts/1 -> HfaMotor_Runnable_sys/62(SysServCarCfgSts_SysServDULin4HFAFSts_read)
  SysServCarCfgSts_SysServDUSFLFootSts/1 -> HfaMotor_Runnable_sys/63(SysServCarCfgSts_SysServDUSFLFootSts_read)
  SysServCarCfgSts_SysServDUSFLVentSts/1 -> HfaMotor_Runnable_sys/64(SysServCarCfgSts_SysServDUSFLVentSts_read)
  SysServCarCfgSts_SysServDUSFRFootSts/1 -> HfaMotor_Runnable_sys/65(SysServCarCfgSts_SysServDUSFRFootSts_read)
  SysServCarCfgSts_SysServDUSFRVentSts/1 -> HfaMotor_Runnable_sys/66(SysServCarCfgSts_SysServDUSFRVentSts_read)
  SysServCarCfgSts_SysServDUSnsrForFCmptmtTSts/1 -> HfaMotor_Runnable_sys/67(SysServCarCfgSts_SysServDUSnsrForFCmptmtTSts_read)
  SysServCarCfgSts_SysServDUSnsrForRair/1 -> HfaMotor_Runnable_sys/68(SysServCarCfgSts_SysServDUSnsrForRair_read)
  SysServCarCfgSts_SysServDUSnsrForRCmptmtTSts/1 -> HfaMotor_Runnable_sys/69(SysServCarCfgSts_SysServDUSnsrForRCmptmtTSts_read)
  BatLoadBtrMSts_BatLoadBtrMSts/1 -> HfaMotor_Runnable_sys/70(BatLoadBtrMSts_BatLoadBtrMSts_read)
  HfaMotSetPowerOnInit_PowerOnInit/1 -> HfaMotor_Runnable_sys/71(HfaMotSetPowerOnInit_PowerOnInit_read)
  VehModMngtGlbSafe1_VehModMngtGlbSafe1/1 -> HfaMotor_Runnable_sys/72(VehModMngtGlbSafe1_VehModMngtGlbSafe1_read)
  HfaActr01ActPson_HfaActr01ActPson/1 -> HfaMotor_Runnable_sys/73(HfaActr01ActPson_HfaActr01ActPson_read)
  HfaActr01ActPson_HfaActr01ActPson_IsUpdated/1 -> HfaMotor_Runnable_sys/74(HfaActr01ActPson_HfaActr01ActPson_IsUpdated_read)
  HfaActr01BlckInd_HfaActr01BlckInd/1 -> HfaMotor_Runnable_sys/75(HfaActr01BlckInd_HfaActr01BlckInd_read)
  HfaActr01BlckSts_HfaActr01BlckSts/1 -> HfaMotor_Runnable_sys/76(HfaActr01BlckSts_HfaActr01BlckSts_read)
  HfaActr01BusAdr_HfaActr01BusAdr/1 -> HfaMotor_Runnable_sys/77(HfaActr01BusAdr_HfaActr01BusAdr_read)
  HfaActr01CoilSts_HfaActr01CoilSts/1 -> HfaMotor_Runnable_sys/78(HfaActr01CoilSts_HfaActr01CoilSts_read)
  HfaActr01DrvDir_HfaActr01DrvDir/1 -> HfaMotor_Runnable_sys/79(HfaActr01DrvDir_HfaActr01DrvDir_read)
  HfaActr01ElecErr_HfaActr01ElecErr/1 -> HfaMotor_Runnable_sys/80(HfaActr01ElecErr_HfaActr01ElecErr_read)
  HfaActr01Mode_HfaActr01Mode/1 -> HfaMotor_Runnable_sys/81(HfaActr01Mode_HfaActr01Mode_read)
  HfaActr01MovDir_HfaActr01MovDir/1 -> HfaMotor_Runnable_sys/82(HfaActr01MovDir_HfaActr01MovDir_read)
  HfaActr01OvrTempErr_HfaActr01OvrTempErr/1 -> HfaMotor_Runnable_sys/83(HfaActr01OvrTempErr_HfaActr01OvrTempErr_read)
  HfaActr01PsonSts_HfaActr01PsonSts/1 -> HfaMotor_Runnable_sys/84(HfaActr01PsonSts_HfaActr01PsonSts_read)
  HfaActr01ResetSts_HfaActr01ResetSts/1 -> HfaMotor_Runnable_sys/85(HfaActr01ResetSts_HfaActr01ResetSts_read)
  HfaActr01SpclFct_HfaActr01SpclFct/1 -> HfaMotor_Runnable_sys/86(HfaActr01SpclFct_HfaActr01SpclFct_read)
  HfaActr01SpdSts_HfaActr01SpdSts/1 -> HfaMotor_Runnable_sys/87(HfaActr01SpdSts_HfaActr01SpdSts_read)
  HfaActr01TrqSts_HfaActr01TrqSts/1 -> HfaMotor_Runnable_sys/88(HfaActr01TrqSts_HfaActr01TrqSts_read)
  HfaActr01UrgcExist_HfaActr01UrgcExist/1 -> HfaMotor_Runnable_sys/89(HfaActr01UrgcExist_HfaActr01UrgcExist_read)
  HfaActr01UrgcPson_HfaActr01UrgcPson/1 -> HfaMotor_Runnable_sys/90(HfaActr01UrgcPson_HfaActr01UrgcPson_read)
  HfaActr01UrgcSts_HfaActr01UrgcSts/1 -> HfaMotor_Runnable_sys/91(HfaActr01UrgcSts_HfaActr01UrgcSts_read)
  HfaActr01VoltErr_HfaActr01VoltErr/1 -> HfaMotor_Runnable_sys/92(HfaActr01VoltErr_HfaActr01VoltErr_read)
  HfaActr02ActPson_HfaActr02ActPson/1 -> HfaMotor_Runnable_sys/93(HfaActr02ActPson_HfaActr02ActPson_read)
  HfaActr02ActPson_HfaActr02ActPson_IsUpdated/1 -> HfaMotor_Runnable_sys/94(HfaActr02ActPson_HfaActr02ActPson_IsUpdated_read)
  HfaActr02BlckInd_HfaActr02BlckInd/1 -> HfaMotor_Runnable_sys/95(HfaActr02BlckInd_HfaActr02BlckInd_read)
  HfaActr02BlckSts_HfaActr02BlckSts/1 -> HfaMotor_Runnable_sys/96(HfaActr02BlckSts_HfaActr02BlckSts_read)
  HfaActr02BusAdr_HfaActr02BusAdr/1 -> HfaMotor_Runnable_sys/97(HfaActr02BusAdr_HfaActr02BusAdr_read)
  HfaActr02CoilSts_HfaActr02CoilSts/1 -> HfaMotor_Runnable_sys/98(HfaActr02CoilSts_HfaActr02CoilSts_read)
  HfaActr02DrvDir_HfaActr02DrvDir/1 -> HfaMotor_Runnable_sys/99(HfaActr02DrvDir_HfaActr02DrvDir_read)
  HfaActr02ElecErr_HfaActr02ElecErr/1 -> HfaMotor_Runnable_sys/100(HfaActr02ElecErr_HfaActr02ElecErr_read)
  HfaActr02Mode_HfaActr02Mode/1 -> HfaMotor_Runnable_sys/101(HfaActr02Mode_HfaActr02Mode_read)
  HfaActr02MovDir_HfaActr02MovDir/1 -> HfaMotor_Runnable_sys/102(HfaActr02MovDir_HfaActr02MovDir_read)
  HfaActr02OvrTempErr_HfaActr02OvrTempErr/1 -> HfaMotor_Runnable_sys/103(HfaActr02OvrTempErr_HfaActr02OvrTempErr_read)
  HfaActr02PsonSts_HfaActr02PsonSts/1 -> HfaMotor_Runnable_sys/104(HfaActr02PsonSts_HfaActr02PsonSts_read)
  HfaActr02ResetSts_HfaActr02ResetSts/1 -> HfaMotor_Runnable_sys/105(HfaActr02ResetSts_HfaActr02ResetSts_read)
  HfaActr02SpclFct_HfaActr02SpclFct/1 -> HfaMotor_Runnable_sys/106(HfaActr02SpclFct_HfaActr02SpclFct_read)
  HfaActr02SpdSts_HfaActr02SpdSts/1 -> HfaMotor_Runnable_sys/107(HfaActr02SpdSts_HfaActr02SpdSts_read)
  HfaActr02TrqSts_HfaActr02TrqSts/1 -> HfaMotor_Runnable_sys/108(HfaActr02TrqSts_HfaActr02TrqSts_read)
  HfaActr02UrgcExist_HfaActr02UrgcExist/1 -> HfaMotor_Runnable_sys/109(HfaActr02UrgcExist_HfaActr02UrgcExist_read)
  HfaActr02UrgcPson_HfaActr02UrgcPson/1 -> HfaMotor_Runnable_sys/110(HfaActr02UrgcPson_HfaActr02UrgcPson_read)
  HfaActr02UrgcSts_HfaActr02UrgcSts/1 -> HfaMotor_Runnable_sys/111(HfaActr02UrgcSts_HfaActr02UrgcSts_read)
  HfaActr02VoltErr_HfaActr02VoltErr/1 -> HfaMotor_Runnable_sys/112(HfaActr02VoltErr_HfaActr02VoltErr_read)
  HfaActr03ActPson_HfaActr03ActPson/1 -> HfaMotor_Runnable_sys/113(HfaActr03ActPson_HfaActr03ActPson_read)
  HfaActr03ActPson_HfaActr03ActPson_IsUpdated/1 -> HfaMotor_Runnable_sys/114(HfaActr03ActPson_HfaActr03ActPson_IsUpdated_read)
  HfaActr03BlckInd_HfaActr03BlckInd/1 -> HfaMotor_Runnable_sys/115(HfaActr03BlckInd_HfaActr03BlckInd_read)
  HfaActr03BlckSts_HfaActr03BlckSts/1 -> HfaMotor_Runnable_sys/116(HfaActr03BlckSts_HfaActr03BlckSts_read)
  HfaActr03BusAdr_HfaActr03BusAdr/1 -> HfaMotor_Runnable_sys/117(HfaActr03BusAdr_HfaActr03BusAdr_read)
  HfaActr03CoilSts_HfaActr03CoilSts/1 -> HfaMotor_Runnable_sys/118(HfaActr03CoilSts_HfaActr03CoilSts_read)
  HfaActr03DrvDir_HfaActr03DrvDir/1 -> HfaMotor_Runnable_sys/119(HfaActr03DrvDir_HfaActr03DrvDir_read)
  HfaActr03ElecErr_HfaActr03ElecErr/1 -> HfaMotor_Runnable_sys/120(HfaActr03ElecErr_HfaActr03ElecErr_read)
  HfaActr03Mode_HfaActr03Mode/1 -> HfaMotor_Runnable_sys/121(HfaActr03Mode_HfaActr03Mode_read)
  HfaActr03MovDir_HfaActr03MovDir/1 -> HfaMotor_Runnable_sys/122(HfaActr03MovDir_HfaActr03MovDir_read)
  HfaActr03OvrTempErr_HfaActr03OvrTempErr/1 -> HfaMotor_Runnable_sys/123(HfaActr03OvrTempErr_HfaActr03OvrTempErr_read)
  HfaActr03PsonSts_HfaActr03PsonSts/1 -> HfaMotor_Runnable_sys/124(HfaActr03PsonSts_HfaActr03PsonSts_read)
  HfaActr03ResetSts_HfaActr03ResetSts/1 -> HfaMotor_Runnable_sys/125(HfaActr03ResetSts_HfaActr03ResetSts_read)
  HfaActr03SpclFct_HfaActr03SpclFct/1 -> HfaMotor_Runnable_sys/126(HfaActr03SpclFct_HfaActr03SpclFct_read)
  HfaActr03SpdSts_HfaActr03SpdSts/1 -> HfaMotor_Runnable_sys/127(HfaActr03SpdSts_HfaActr03SpdSts_read)
  HfaActr03TrqSts_HfaActr03TrqSts/1 -> HfaMotor_Runnable_sys/128(HfaActr03TrqSts_HfaActr03TrqSts_read)
  HfaActr03UrgcExist_HfaActr03UrgcExist/1 -> HfaMotor_Runnable_sys/129(HfaActr03UrgcExist_HfaActr03UrgcExist_read)
  HfaActr03UrgcPson_HfaActr03UrgcPson/1 -> HfaMotor_Runnable_sys/130(HfaActr03UrgcPson_HfaActr03UrgcPson_read)
  HfaActr03UrgcSts_HfaActr03UrgcSts/1 -> HfaMotor_Runnable_sys/131(HfaActr03UrgcSts_HfaActr03UrgcSts_read)
  HfaActr03VoltErr_HfaActr03VoltErr/1 -> HfaMotor_Runnable_sys/132(HfaActr03VoltErr_HfaActr03VoltErr_read)
  HfaActr04ActPson_HfaActr04ActPson/1 -> HfaMotor_Runnable_sys/133(HfaActr04ActPson_HfaActr04ActPson_read)
  HfaActr04ActPson_HfaActr04ActPson_IsUpdated/1 -> HfaMotor_Runnable_sys/134(HfaActr04ActPson_HfaActr04ActPson_IsUpdated_read)
  HfaActr04BlckInd_HfaActr04BlckInd/1 -> HfaMotor_Runnable_sys/135(HfaActr04BlckInd_HfaActr04BlckInd_read)
  HfaActr04BlckSts_HfaActr04BlckSts/1 -> HfaMotor_Runnable_sys/136(HfaActr04BlckSts_HfaActr04BlckSts_read)
  HfaActr04BusAdr_HfaActr04BusAdr/1 -> HfaMotor_Runnable_sys/137(HfaActr04BusAdr_HfaActr04BusAdr_read)
  HfaActr04CoilSts_HfaActr04CoilSts/1 -> HfaMotor_Runnable_sys/138(HfaActr04CoilSts_HfaActr04CoilSts_read)
  HfaActr04DrvDir_HfaActr04DrvDir/1 -> HfaMotor_Runnable_sys/139(HfaActr04DrvDir_HfaActr04DrvDir_read)
  HfaActr04ElecErr_HfaActr04ElecErr/1 -> HfaMotor_Runnable_sys/140(HfaActr04ElecErr_HfaActr04ElecErr_read)
  HfaActr04Mode_HfaActr04Mode/1 -> HfaMotor_Runnable_sys/141(HfaActr04Mode_HfaActr04Mode_read)
  HfaActr04MovDir_HfaActr04MovDir/1 -> HfaMotor_Runnable_sys/142(HfaActr04MovDir_HfaActr04MovDir_read)
  HfaActr04OvrTempErr_HfaActr04OvrTempErr/1 -> HfaMotor_Runnable_sys/143(HfaActr04OvrTempErr_HfaActr04OvrTempErr_read)
  HfaActr04PsonSts_HfaActr04PsonSts/1 -> HfaMotor_Runnable_sys/144(HfaActr04PsonSts_HfaActr04PsonSts_read)
  HfaActr04ResetSts_HfaActr04ResetSts/1 -> HfaMotor_Runnable_sys/145(HfaActr04ResetSts_HfaActr04ResetSts_read)
  HfaActr04SpclFct_HfaActr04SpclFct/1 -> HfaMotor_Runnable_sys/146(HfaActr04SpclFct_HfaActr04SpclFct_read)
  HfaActr04SpdSts_HfaActr04SpdSts/1 -> HfaMotor_Runnable_sys/147(HfaActr04SpdSts_HfaActr04SpdSts_read)
  HfaActr04TrqSts_HfaActr04TrqSts/1 -> HfaMotor_Runnable_sys/148(HfaActr04TrqSts_HfaActr04TrqSts_read)
  HfaActr04UrgcExist_HfaActr04UrgcExist/1 -> HfaMotor_Runnable_sys/149(HfaActr04UrgcExist_HfaActr04UrgcExist_read)
  HfaActr04UrgcPson_HfaActr04UrgcPson/1 -> HfaMotor_Runnable_sys/150(HfaActr04UrgcPson_HfaActr04UrgcPson_read)
  HfaActr04UrgcSts_HfaActr04UrgcSts/1 -> HfaMotor_Runnable_sys/151(HfaActr04UrgcSts_HfaActr04UrgcSts_read)
  HfaActr04VoltErr_HfaActr04VoltErr/1 -> HfaMotor_Runnable_sys/152(HfaActr04VoltErr_HfaActr04VoltErr_read)
  HfaActr05ActPson_HfaActr05ActPson/1 -> HfaMotor_Runnable_sys/153(HfaActr05ActPson_HfaActr05ActPson_read)
  HfaActr05ActPson_HfaActr05ActPson_IsUpdated/1 -> HfaMotor_Runnable_sys/154(HfaActr05ActPson_HfaActr05ActPson_IsUpdated_read)
  HfaActr05BlckInd_HfaActr05BlckInd/1 -> HfaMotor_Runnable_sys/155(HfaActr05BlckInd_HfaActr05BlckInd_read)
  HfaActr05BlckSts_HfaActr05BlckSts/1 -> HfaMotor_Runnable_sys/156(HfaActr05BlckSts_HfaActr05BlckSts_read)
  HfaActr05BusAdr_HfaActr05BusAdr/1 -> HfaMotor_Runnable_sys/157(HfaActr05BusAdr_HfaActr05BusAdr_read)
  HfaActr05CoilSts_HfaActr05CoilSts/1 -> HfaMotor_Runnable_sys/158(HfaActr05CoilSts_HfaActr05CoilSts_read)
  HfaActr05DrvDir_HfaActr05DrvDir/1 -> HfaMotor_Runnable_sys/159(HfaActr05DrvDir_HfaActr05DrvDir_read)
  HfaActr05ElecErr_HfaActr05ElecErr/1 -> HfaMotor_Runnable_sys/160(HfaActr05ElecErr_HfaActr05ElecErr_read)
  HfaActr05Mode_HfaActr05Mode/1 -> HfaMotor_Runnable_sys/161(HfaActr05Mode_HfaActr05Mode_read)
  HfaActr05MovDir_HfaActr05MovDir/1 -> HfaMotor_Runnable_sys/162(HfaActr05MovDir_HfaActr05MovDir_read)
  HfaActr05OvrTempErr_HfaActr05OvrTempErr/1 -> HfaMotor_Runnable_sys/163(HfaActr05OvrTempErr_HfaActr05OvrTempErr_read)
  HfaActr05PsonSts_HfaActr05PsonSts/1 -> HfaMotor_Runnable_sys/164(HfaActr05PsonSts_HfaActr05PsonSts_read)
  HfaActr05ResetSts_HfaActr05ResetSts/1 -> HfaMotor_Runnable_sys/165(HfaActr05ResetSts_HfaActr05ResetSts_read)
  HfaActr05SpclFct_HfaActr05SpclFct/1 -> HfaMotor_Runnable_sys/166(HfaActr05SpclFct_HfaActr05SpclFct_read)
  HfaActr05SpdSts_HfaActr05SpdSts/1 -> HfaMotor_Runnable_sys/167(HfaActr05SpdSts_HfaActr05SpdSts_read)
  HfaActr05TrqSts_HfaActr05TrqSts/1 -> HfaMotor_Runnable_sys/168(HfaActr05TrqSts_HfaActr05TrqSts_read)
  HfaActr05UrgcExist_HfaActr05UrgcExist/1 -> HfaMotor_Runnable_sys/169(HfaActr05UrgcExist_HfaActr05UrgcExist_read)
  HfaActr05UrgcPson_HfaActr05UrgcPson/1 -> HfaMotor_Runnable_sys/170(HfaActr05UrgcPson_HfaActr05UrgcPson_read)